simulink model "ofdm_txrx_supermimo"  (Simulink 7.1)
settings: Solver=VariableStepDiscrete  SolverMode=SingleTasking  StartTime=0.0  StopTime=simOnly_simLength  RelTol=1e-3
PostLoadFcn:
  | ofdm_rx_supermimo_init
InitFcn:
  | ofdm_rx_supermimo_init
StartFcn:
  | ofdm_rx_supermimo_init
values from neighbouring files (symbols this model uses):
  Action0_Config = 0* 31*2^18 + 0*2^24 + PHY_AUTORESPONSE_REQ_GOODHDR + PHY_AUTORESPONSE_REQ_GOODPKT + PHY_AUTORESPONSE_REQ_FLAGA    [ofdm_tx_supermimo_init.m]
  Action1_Config = 0* 62*2^18 + PHY_AUTORESPONSE_REQ_GOODHDR + PHY_AUTORESPONSE_REQ_GOODPKT    [ofdm_tx_supermimo_init.m]
  Action2_Config = 0    [ofdm_tx_supermimo_init.m]
  Action3_Config = 0    [ofdm_tx_supermimo_init.m]
  Action4_Config = 0    [ofdm_tx_supermimo_init.m]
  Action5_Config = 0    [ofdm_tx_supermimo_init.m]
  ActionID_AFTransmit = 31    [ofdm_tx_supermimo_init.m]
  ActionID_Disabled = 0    [ofdm_tx_supermimo_init.m]
  ActionID_SetFlagA = 62    [ofdm_tx_supermimo_init.m]
  ActionID_SetFlagB = 61    [ofdm_tx_supermimo_init.m]
  BER_RAM_init_size = 2048    [ofdm_tx_supermimo_init.m]
  CPLength = 16    [ofdm_tx_supermimo_init.m]
  longCorr_coef_i = [-4*real(LTS) 0]    [ofdm_rx_supermimo_init.m]
  longCorr_coef_q = [4*imag(LTS) 0]    [ofdm_rx_supermimo_init.m]
  Match0_Config = 0    [ofdm_tx_supermimo_init.m]
  Match1_Config = 0    [ofdm_tx_supermimo_init.m]
  Match2_Config = 0    [ofdm_tx_supermimo_init.m]
  Match3_Config = 0    [ofdm_tx_supermimo_init.m]
  Match4_Config = 0    [ofdm_tx_supermimo_init.m]
  Match5_Config = 0    [ofdm_tx_supermimo_init.m]
  Match6_Config = 0    [ofdm_tx_supermimo_init.m]
  Match7_Config = 0    [ofdm_tx_supermimo_init.m]
  max_CP_length = 16    [ofdm_tx_supermimo_init.m]
  max_num_baseRateSymbols = 31    [ofdm_tx_supermimo_init.m]
  max_num_subcarriers = 64    [ofdm_tx_supermimo_init.m]
  max_num_trainingSymbols = 15    [ofdm_tx_supermimo_init.m]
  max_numBytes = 16384    [ofdm_tx_supermimo_init.m]
  max_OFDM_symbols = 511    [ofdm_tx_supermimo_init.m]
  modConstellation_qam16 = .75*[1 3 -1 -3]./3    [ofdm_tx_supermimo_init.m]
  modConstellation_qam64 = 0.875*[3 1 5 7 -3 -1 -5 -7]./7    [ofdm_tx_supermimo_init.m]
  modConstellation_qpsk = [1 -1]./sqrt(2)    [ofdm_tx_supermimo_init.m]
  numBaseRateSymbols = ceil(numHeaderBytes / numBytes_BaseRateOFDMSymbol)    [ofdm_tx_supermimo_init.m]
  numSubcarriers = 64    [ofdm_tx_supermimo_init.m]
  numTrainingSymbols = 2    [ofdm_tx_supermimo_init.m]
  pilot_indicies = 7 + ( (64-21) * 2^8) + ( (64-7) * 2^16) + (21 * 2^24)    (= 356068103)    [ofdm_tx_supermimo_init.m]
  pilotValues = hex2dec('A57D') + (2^16 * hex2dec('5A82'))    [ofdm_tx_supermimo_init.m]
  pkt_crossCorr_thresh = 0.7    [ofdm_rx_supermimo_init.m]
  pkt_energy_thresh = 0    [ofdm_rx_supermimo_init.m]
  pktByteNums = numHeaderBytes +  				byteIndex_numPayloadBytes(1)*2^8 +  				byteIndex_numPayloadBytes(2)*2^16 +  				byteIndex_simpleDynModMasks * 2^24    [ofdm_rx_supermimo_init.m]
  PktDet_Delay = 58+32 +      Symbol_Timing_Offset*2^7 +      0*2^16 +      (117* 2^24)    (= 1962935642)    [ofdm_rx_supermimo_init.m]
  pktTiming_count_payloadStart = pktTiming_count_lsStart + pktTiming_ls_num*pktTiming_ls_length    [ofdm_rx_supermimo_init.m]
  preamble = preamble_scale*[shortsyms_10 longsyms_2]    [ofdm_tx_supermimo_init.m]
  preamble_ROM = preamble_I_ROM + preamble_Q_ROM*2^16    [ofdm_tx_supermimo_init.m]
  RAM_init_size = max_numBytes    (= 16384)    [ofdm_tx_supermimo_init.m]
  reg_AF_Tx_Blanking = (320+80) + ( (320+80+79)*2^16)    (= 31392144)    [ofdm_rx_supermimo_init.m]
  reg_AF_Tx_Scaling = 2944    [ofdm_rx_supermimo_init.m]
  reg_Interrupt_PktBuf_Control = reg_InterruptControl +  	1 * 2^16 +  	0 * 2^24    (= 65656)    [ofdm_rx_supermimo_init.m]
  reg_PilotCalcParams = (4 * 2^16) + (minPilotChanMag)    (= 262184.96)    [ofdm_rx_supermimo_init.m]
  regRx_chanEst_minMag = ((0.5)*2^15) + ((0.5 * 2^15)*2^16)    (= 1073758208)    [ofdm_rx_supermimo_init.m]
  regRx_coarseCalcCorrection = round((1.3e-3 - 1.2982e-3)*2^32)    [ofdm_rx_supermimo_init.m]
  regRx_pilotCalcCorrection = round(1.00115 * 2^31)    [ofdm_rx_supermimo_init.m]
  regRx_preCFOoptions = 0 * 2^0 +  	1 * 2^1 +  	0    (= 2)    [ofdm_rx_supermimo_init.m]
  rx_controlBits = 1 * 2  		 + 1 * 4  		 + 0 * 8  		 + 1 * 16 * rx_SISO_Mode  		 + 1 * 32  		 + 1 * 64  		 + 1 * 128  		 + 1 * 256  		 + 1 * 512  		 + 1 * 1024  		 + 1 * 4096  		 + 0 * 16384  		 + 0 * 32768  		 + 1 * 65536  		 + 0 * 131072  		 + 0 * 262144  		 + 1 * 524288  		 + 1 * 1048576 * tx_Alamouti_Mode  		 + 0 * 2097152           + 1 * 33554432  		 + 0 * 4194304  		 + 0 * 8388608  		 + 0 * 16777216  		 + 1 *  ...(+49 chars)    [ofdm_rx_supermimo_init.m]
  Rx_PktDet_LongCorr_Params = LongCorrCounterLoad + 0*2^8 + 0*2^16 + 255*2^24    (= 4278190325)    [ofdm_rx_supermimo_init.m]
  Rx_PktDet_LongCorrThresholds = 8000    [ofdm_rx_supermimo_init.m]
  rx_postEq_scaling = round(rxScaling*2^11) * (1 + 2^16)    [ofdm_rx_supermimo_init.m]
  RxDataScrambling_Seq = [40 198 78 63 82 173 102 245 48 111 172 115 147 230 216 93 72 65 62 2 205 242 122 90 128 83 105 97 73 10 5 252]    [ofdm_tx_supermimo_init.m]
  RxReg_FixedPktLen = 0    [ofdm_rx_supermimo_init.m]
  shortSymbol_time = shortSymbol_time(1:16).'    [ofdm_tx_supermimo_init.m]
  simOnly_numSamples = length(preamble)+( (numSubcarriers+CPLength)*(numTrainingSymbols + numBaseRateSymbols + numFullRateSymbols/2) )    [ofdm_tx_supermimo_init.m]
  train = [train train]    [ofdm_tx_supermimo_init.m]
  tx_controlBits = (antB_preambleShift * 2^4) + (2^1 * tx_Alamouti_Mode) +  	(2^0 * tx_SISO_Mode) + 4*0 + 8*1 + 0*256 + 512*0 + 1024*0 +  	0*hex2dec('1000') + 0*hex2dec('2000') + 0*hex2dec('4000') +  	1*hex2dec('8000') + 1*hex2dec('10000') + hex2dec('20000') +  	16*hex2dec('100000')    [ofdm_tx_supermimo_init.m]
  tx_delays = (50*2^0) + (0*2^8) + (30*2^12)    (= 122930)    [ofdm_tx_supermimo_init.m]
  tx_scaling = (3072) + (12288 * 2^16)    (= 805309440)    [ofdm_tx_supermimo_init.m]
  TxDataScrambling_Seq = [40 198 78 63 82 173 102 245 48 111 172 115 147 230 216 93 72 65 62 2 205 242 122 90 128 83 105 97 73 10 5 252]    [ofdm_tx_supermimo_init.m]
  txfilt_firpm = 0.95*txfilt_firpm./(max(txfilt_firpm))    [ofdm_tx_supermimo_init.m]
  txfilt_intfilt = [0 intfilt(4,4,.8)]    [ofdm_tx_supermimo_init.m]
  txReg_symbolCounts = (2^8 *numBaseRateSymbols ) + numTrainingSymbols    [ofdm_tx_supermimo_init.m]
  TxRx_FFTScaling = (3 * 2^0) +  	(2 * 2^2) +  	(1 * 2^4) +  	(1 * 2^6) +  	(1 * 2^8) +  	(0 * 2^10)    (= 347)    [ofdm_tx_supermimo_init.m]
undefined symbols (not defined in the file or in its folder): AntA, AntB, BaseRate, Down, gcbh, numBuffs, numBytes, Up, xlBlockLoadCallback, xlGetSimulinkPeriod, xlipgetpartsetting
libraries: xbsIndex_r4 x6035, simulink x4, dspsigops x4, commchan3 x2, dspsrcs4 x2, commrflib2 x2
(part 5 of 61)

== system ofdm_txrx_supermimo/Memory-mapped Registers/Static Parameters (might be registers someday) ==
  note: Replace with registers someday
      | to support dynamic transform and
      | cyclic prefix sizes
  Constant : xbsIndex_r4/Constant  arith_type=Unsigned  const=numSubcarriers  n_bits=ceil(log2(max_num_subcarriers)+1)  bin_pt=0  explicit_period=on  period=2  equ=P=C  opselect=C  inp2=PCIN>>17  opr=+  inp1=P  carry=CIN  dbl_ovrd=off  block_type=constant
      settings: block_version=VER_STRING_GOES_HERE
  Constant1 : xbsIndex_r4/Constant  arith_type=Unsigned  const=numSubcarriers+CPLength-1  n_bits=ceil(log2(max_num_subcarriers+CPLength)+1)  bin_pt=0  explicit_period=on  period=2  equ=P=C  opselect=C  inp2=PCIN>>17  opr=+  inp1=P  carry=CIN  dbl_ovrd=off  block_type=constant
      settings: block_version=VER_STRING_GOES_HERE
  Constant2 : xbsIndex_r4/Constant  arith_type=Unsigned  const=16  n_bits=5  bin_pt=0  explicit_period=on  period=1  equ=P=C  opselect=C  inp2=PCIN>>17  opr=+  inp1=P  carry=CIN  dbl_ovrd=off  block_type=constant
      settings: block_version=VER_STRING_GOES_HERE
  Down Sample8 : xbsIndex_r4/Down Sample  sample_ratio=2  sample_phase="Last Value of Frame  (most efficient)"  en=off  latency=1  dbl_ovrd=off  block_type=dsamp
      settings: block_version=VER_STRING_GOES_HERE
  tag scope: regRX_CP_Length=global, regRX_numSubcarriers=global, regRX_sampsPerSymMinusOne=global
  wires:
  Constant/1 -> [regRX_numSubcarriers]
  Constant1/1 -> [regRX_sampsPerSymMinusOne]
  Down Sample8/1 -> [regRX_CP_Length]
  Constant2/1 -> Down Sample8/1

== system ofdm_txrx_supermimo/Memory-mapped Registers/Tx Registers ==
  note: 0x2
  note: 0x1
  note: 0x0000_00FF - 8 bits
  note: 0x0000_0F00 - 4 bits
  note: 0x000F_F000 - 8 bits
  Control Bits Slices : Subsystem
  Down Sample1 : xbsIndex_r4/Down Sample  sample_ratio=2  sample_phase="Last Value of Frame  (most efficient)"  en=off  latency=1  dbl_ovrd=off  block_type=dsamp
      settings: block_version=VER_STRING_GOES_HERE
  Down Sample3 : xbsIndex_r4/Down Sample  sample_ratio=2  sample_phase="Last Value of Frame  (most efficient)"  en=off  latency=1  dbl_ovrd=off  block_type=dsamp
      settings: block_version=VER_STRING_GOES_HERE
  Down Sample4 : xbsIndex_r4/Down Sample  sample_ratio=4  sample_phase="Last Value of Frame  (most efficient)"  en=off  latency=1  dbl_ovrd=off  block_type=dsamp
      settings: block_version=VER_STRING_GOES_HERE
  Down Sample6 : xbsIndex_r4/Down Sample  sample_ratio=2  sample_phase="Last Value of Frame  (most efficient)"  en=off  latency=1  dbl_ovrd=off  block_type=dsamp
      settings: block_version=VER_STRING_GOES_HERE
  Read Only Register : Subsystem  AncestorBlock=|  mask{regName='Tx_PktRunning'}
      | WARP_Blockset/Register Wrappers
      | (Sysgen 10.1+)/Read Only Register
      mask prompts: regName "Register Name"
      mask help:
      | This block is a wrapper for System Generator's To Register shared memory block. This register is designed for use with WARP's sysgen2opb tool.
      |
      | Note: The Regisger Name paramter below must be unique for every instance of Read-Write and Read-Only registers in your system.
      mask init: same as ofdm_txrx_supermimo/Memory-mapped Registers/Rx Registers/Read Only Register
      settings: AttributesFormatString=RO Reg: %<regName>\n%<ReferenceBlock>
  Read-Write Register : Subsystem  AncestorBlock=|  mask{regName='Tx_Start_Reset_Control', out_dataType=Unsigned, out_numBits=32, out_binaryPt=0, initValue=0, sampPeriod=1}
      | WARP_Blockset/Register Wrappers
      | (Sysgen 10.1+)/Read-Write Register
      mask prompts: regName "Register Name"; out_dataType "Output Type"; out_numBits "Output Num Bits"; out_binaryPt "Output Binary Point"; initValue "Initial Value"; sampPeriod "Sample Period"
      mask help:
      | This block is a wrapper for two registers from the System Generator Shared Memory blockset:
      | a) A "From Register" block, which provides a write-only register for the host processor
      | b) A "To Register" block, which provides a read-only register for the host processor
      |
      | Note: The Regisger Name paramter below must be unique for every instance of shared-memory registers in your system. System Generator will throw an error if duplicate names are found.
      mask init: same as ofdm_txrx_supermimo/Memory-mapped Registers/AutoReply Registers/Read-Write Register1
      settings: AttributesFormatString=RW Reg: %<regName>
      mask StyleString: edit,popup(Unsigned|Signed  (2's comp)),edit,edit,edit,edit
  Read-Write Register1 : Subsystem  AncestorBlock=|  mask{regName='Tx_ControlBits', out_dataType=Unsigned, out_numBits=32, out_binaryPt=0, initValue=tx_controlBits, sampPeriod=1}
      | WARP_Blockset/Register Wrappers
      | (Sysgen 10.1+)/Read-Write Register
      mask prompts: regName "Register Name"; out_dataType "Output Type"; out_numBits "Output Num Bits"; out_binaryPt "Output Binary Point"; initValue "Initial Value"; sampPeriod "Sample Period"
      mask help:
      | This block is a wrapper for two registers from the System Generator Shared Memory blockset:
      | a) A "From Register" block, which provides a write-only register for the host processor
      | b) A "To Register" block, which provides a read-only register for the host processor
      |
      | Note: The Regisger Name paramter below must be unique for every instance of shared-memory registers in your system. System Generator will throw an error if duplicate names are found.
      mask init: same as ofdm_txrx_supermimo/Memory-mapped Registers/AutoReply Registers/Read-Write Register1
      settings: AttributesFormatString=RW Reg: %<regName>
      mask StyleString: edit,popup(Unsigned|Signed  (2's comp)),edit,edit,edit,edit
  Read-Write Register2 : Subsystem  AncestorBlock=|  mask{regName='Tx_Delays', out_dataType=Unsigned, out_numBits=32, out_binaryPt=0, initValue=tx_delays, sampPeriod=1}
      | WARP_Blockset/Register Wrappers
      | (Sysgen 10.1+)/Read-Write Register
      mask prompts: regName "Register Name"; out_dataType "Output Type"; out_numBits "Output Num Bits"; out_binaryPt "Output Binary Point"; initValue "Initial Value"; sampPeriod "Sample Period"
      mask help:
      | This block is a wrapper for two registers from the System Generator Shared Memory blockset:
      | a) A "From Register" block, which provides a write-only register for the host processor
      | b) A "To Register" block, which provides a read-only register for the host processor
      |
      | Note: The Regisger Name paramter below must be unique for every instance of shared-memory registers in your system. System Generator will throw an error if duplicate names are found.
      mask init: same as ofdm_txrx_supermimo/Memory-mapped Registers/AutoReply Registers/Read-Write Register1
      settings: AttributesFormatString=RW Reg: %<regName>
      mask StyleString: edit,popup(Unsigned|Signed  (2's comp)),edit,edit,edit,edit
  Reinterpret : xbsIndex_r4/Reinterpret  force_arith_type=on  arith_type=Unsigned  force_bin_pt=on  bin_pt=13  block_type=reinterpret
      settings: infoedit="Changes signal type without altering the binary representation.   You can changed the signal between signed and unsigned, and relocate the binary point.<P><P>Hardware notes: In hardware this block costs nothing.<P><P>Example:  Suppose the input is 6 bits wide, signed, with 2 fractional bits, and the output is forced to unsigned with 0 fractional bits.  Then an input of -2.0 (1110.00 in binary 2's complement) becomes an output of 56 (111000 in binary)."  block_version=10.1
  Reinterpret1 : xbsIndex_r4/Reinterpret  force_arith_type=on  arith_type=Unsigned  force_bin_pt=on  bin_pt=13  block_type=reinterpret
      settings: infoedit="Changes signal type without altering the binary representation.   You can changed the signal between signed and unsigned, and relocate the binary point.<P><P>Hardware notes: In hardware this block costs nothing.<P><P>Example:  Suppose the input is 6 bits wide, signed, with 2 fractional bits, and the output is forced to unsigned with 0 fractional bits.  Then an input of -2.0 (1110.00 in binary 2's complement) becomes an output of 56 (111000 in binary)."  block_version=10.1
  Slice1 : xbsIndex_r4/Slice  nbits=4  boolean_output=off  mode=Lower Bit Location + Width  bit1=0  base1=MSB of Input  bit0=8  base0=LSB of Input  dbl_ovrd=off  block_type=slice
      settings: infoedit="Extracts a given range of bits from each input sample and presents it at the output.  The output type is ordinarily unsigned with binary point at zero, but can be Boolean when the slice is one bit wide.<P><P>Hardware notes: In hardware this block costs nothing."  block_version=VER_STRING_GOES_HERE
  Slice10 : xbsIndex_r4/Slice  nbits=8  boolean_output=off  mode=Lower Bit Location + Width  bit1=0  base1=MSB of Input  bit0=0  base0=LSB of Input  dbl_ovrd=off  block_type=slice
      settings: infoedit="Extracts a given range of bits from each input sample and presents it at the output.  The output type is ordinarily unsigned with binary point at zero, but can be Boolean when the slice is one bit wide.<P><P>Hardware notes: In hardware this block costs nothing."  block_version=VER_STRING_GOES_HERE
  Slice14 : xbsIndex_r4/Slice  nbits=1  boolean_output=off  mode=Lower Bit Location + Width  bit1=0  base1=MSB of Input  bit0=0  base0=LSB of Input  dbl_ovrd=off  block_type=slice
      settings: infoedit="Extracts a given range of bits from each input sample and presents it at the output.  The output type is ordinarily unsigned with binary point at zero, but can be Boolean when the slice is one bit wide.<P><P>Hardware notes: In hardware this block costs nothing."  block_version=VER_STRING_GOES_HERE
  Slice15 : xbsIndex_r4/Slice  nbits=1  boolean_output=on  mode=Lower Bit Location + Width  bit1=0  base1=MSB of Input  bit0=1  base0=LSB of Input  dbl_ovrd=off  block_type=slice
      settings: infoedit="Extracts a given range of bits from each input sample and presents it at the output.  The output type is ordinarily unsigned with binary point at zero, but can be Boolean when the slice is one bit wide.<P><P>Hardware notes: In hardware this block costs nothing."  block_version=VER_STRING_GOES_HERE
  Slice2 : xbsIndex_r4/Slice  nbits=8  boolean_output=off  mode=Lower Bit Location + Width  bit1=0  base1=MSB of Input  bit0=12  base0=LSB of Input  dbl_ovrd=off  block_type=slice
      settings: infoedit="Extracts a given range of bits from each input sample and presents it at the output.  The output type is ordinarily unsigned with binary point at zero, but can be Boolean when the slice is one bit wide.<P><P>Hardware notes: In hardware this block costs nothing."  block_version=VER_STRING_GOES_HERE
  Slice7 : xbsIndex_r4/Slice  nbits=16  boolean_output=off  mode=Lower Bit Location + Width  bit1=0  base1=MSB of Input  bit0=0  base0=LSB of Input  dbl_ovrd=off  block_type=slice
      settings: infoedit="Extracts a given range of bits from each input sample and presents it at the output.  The output type is ordinarily unsigned with binary point at zero, but can be Boolean when the slice is one bit wide.<P><P>Hardware notes: In hardware this block costs nothing."  block_version=VER_STRING_GOES_HERE
  Slice8 : xbsIndex_r4/Slice  nbits=16  boolean_output=off  mode=Lower Bit Location + Width  bit1=0  base1=MSB of Input  bit0=16  base0=LSB of Input  dbl_ovrd=off  block_type=slice
      settings: infoedit="Extracts a given range of bits from each input sample and presents it at the output.  The output type is ordinarily unsigned with binary point at zero, but can be Boolean when the slice is one bit wide.<P><P>Hardware notes: In hardware this block costs nothing."  block_version=VER_STRING_GOES_HERE
  Write-Only Register1 : Subsystem  AncestorBlock=|  mask{regName='Tx_Scaling', out_dataType=Unsigned, out_numBits=32, out_binaryPt=0, initValue=tx_scaling, sampPeriod=1}
      | WARP_Blockset/Register Wrappers
      | (Sysgen 10.1+)/Write-Only Register
      mask prompts: regName "Register Name"; out_dataType "Output Type"; out_numBits "Output Num Bits"; out_binaryPt "Output Binary Point"; initValue "Initial Value"; sampPeriod "Sample Period"
      mask help:
      | This block is a wrapper for System Generator's "From Register" shared memory block.
      |
      | Note: The Regisger Name paramter below must be unique for every instance of shared-memory registers in your system. System Generator will throw an error if duplicate names are found.
      mask init: same as ofdm_txrx_supermimo/Memory-mapped Registers/Rx Registers/Write-Only Register
      settings: AttributesFormatString=RW Reg: %<regName>
      mask StyleString: edit,popup(Unsigned|Signed  (2's comp)),edit,edit,edit,edit
  tag scope: TxReg_ExtAutoTxEn_Delay=global, TxReg_PreambleScaling=global, TxReg_TxReset=global, TxReg_TxRunningOut_delay=global, TxReg_TxStart=global, TxReg_postIFFTScaling=global, regRx_AutoTx_ExtraDly=global
  wires:
  Read-Write Register1/1(D) -> Down Sample1/1
  Slice14/1 -> [TxReg_TxReset]
  Read-Write Register/1(D) -> Down Sample3/1
  Slice15/1 -> [TxReg_TxStart]
  Down Sample3/1 -> Slice14/1, Slice15/1
  [regTx_PktRunningEn] -> Read Only Register/2(En)
  Write-Only Register1/1(D) -> Slice8/1, Slice7/1
  Reinterpret/1 -> [TxReg_PreambleScaling]
  Slice7/1 -> Reinterpret/1
  Reinterpret1/1 -> [TxReg_postIFFTScaling]
  Slice8/1 -> Reinterpret1/1
  Down Sample1/1 -> Control Bits Slices/1(32b)
  [regTx_PktRunning] -> Read Only Register/1(D)
  Slice10/1 -> [TxReg_ExtAutoTxEn_Delay]
  Slice1/1 -> [regRx_AutoTx_ExtraDly]
  Slice2/1 -> [TxReg_TxRunningOut_delay]
  Read-Write Register2/1(D) -> Down Sample6/1, Down Sample4/1
  Down Sample6/1 -> Slice1/1, Slice10/1
  Down Sample4/1 -> Slice2/1

== system ofdm_txrx_supermimo/Memory-mapped Registers/TxRx Registers ==
  16LSB+8 : xbsIndex_r4/Slice  nbits=16  boolean_output=off  mode=Lower Bit Location + Width  bit1=0  base1=MSB of Input  bit0=8  base0=LSB of Input  dbl_ovrd=off  block_type=slice
      settings: infoedit="Extracts a given range of bits from each input sample and presents it at the output.  The output type is ordinarily unsigned with binary point at zero, but can be Boolean when the slice is one bit wide.<P><P>Hardware notes: In hardware this block costs nothing."  block_version=VER_STRING_GOES_HERE
  16LSB1 : xbsIndex_r4/Slice  nbits=ceil(log2(max_num_trainingSymbols))  boolean_output=off  mode=Lower Bit Location + Width  bit1=0  base1=MSB of Input  bit0=0  base0=LSB of Input  dbl_ovrd=off  block_type=slice
      settings: infoedit="Extracts a given range of bits from each input sample and presents it at the output.  The output type is ordinarily unsigned with binary point at zero, but can be Boolean when the slice is one bit wide.<P><P>Hardware notes: In hardware this block costs nothing."  block_version=VER_STRING_GOES_HERE
  16MSB1 : xbsIndex_r4/Slice  nbits=ceil(log2(max_num_baseRateSymbols))  boolean_output=off  mode=Lower Bit Location + Width  bit1=0  base1=MSB of Input  bit0=16  base0=LSB of Input  dbl_ovrd=off  block_type=slice
      settings: infoedit="Extracts a given range of bits from each input sample and presents it at the output.  The output type is ordinarily unsigned with binary point at zero, but can be Boolean when the slice is one bit wide.<P><P>Hardware notes: In hardware this block costs nothing."  block_version=VER_STRING_GOES_HERE
  6LSB+6 : xbsIndex_r4/Slice  nbits=6  boolean_output=off  mode=Lower Bit Location + Width  bit1=0  base1=MSB of Input  bit0=6  base0=LSB of Input  dbl_ovrd=off  block_type=slice
      settings: infoedit="Extracts a given range of bits from each input sample and presents it at the output.  The output type is ordinarily unsigned with binary point at zero, but can be Boolean when the slice is one bit wide.<P><P>Hardware notes: In hardware this block costs nothing."  block_version=VER_STRING_GOES_HERE
  6LSB1 : xbsIndex_r4/Slice  nbits=6  boolean_output=off  mode=Lower Bit Location + Width  bit1=0  base1=MSB of Input  bit0=0  base0=LSB of Input  dbl_ovrd=off  block_type=slice
      settings: infoedit="Extracts a given range of bits from each input sample and presents it at the output.  The output type is ordinarily unsigned with binary point at zero, but can be Boolean when the slice is one bit wide.<P><P>Hardware notes: In hardware this block costs nothing."  block_version=VER_STRING_GOES_HERE
  8LSB : xbsIndex_r4/Slice  nbits=8  boolean_output=off  mode=Lower Bit Location + Width  bit1=0  base1=MSB of Input  bit0=0  base0=LSB of Input  dbl_ovrd=off  block_type=slice
      settings: infoedit="Extracts a given range of bits from each input sample and presents it at the output.  The output type is ordinarily unsigned with binary point at zero, but can be Boolean when the slice is one bit wide.<P><P>Hardware notes: In hardware this block costs nothing."  block_version=VER_STRING_GOES_HERE
  AddSub1 : xbsIndex_r4/AddSub  mode=Addition  use_carryin=off  use_carryout=off  en=off  latency=0  precision=User Defined  arith_type=Unsigned  n_bits=1 + ceil(log2(max_num_trainingSymbols + max_num_baseRateSymbols))  bin_pt=0  quantization=Truncate  overflow=Wrap  dbl_ovrd=off  use_behavioral_HDL=on  pipelined=off  use_rpm=on  hw_selection=Fabric  block_type=addsub
      settings: xl_area=[0, 0, 0, 0, 0, 0, 0]  block_version=VER_STRING_GOES_HERE
  Down Sample{4,7,11,25} (4 alike) : xbsIndex_r4/Down Sample  sample_ratio=2  sample_phase="Last Value of Frame  (most efficient)"  en=off  latency=1  dbl_ovrd=off  block_type=dsamp
      settings: block_version=VER_STRING_GOES_HERE
  InterruptControl : Subsystem
  Read-Write Register : Subsystem  AncestorBlock=|  mask{regName='TxRx_Interrupt_PktBuf_Ctrl', out_dataType=Unsigned, out_numBits=32, out_binaryPt=0, initValue=reg_Interrupt_PktBuf_Control, sampPeriod=1}
      | WARP_Blockset/Register Wrappers
      | (Sysgen 10.1+)/Read-Write Register
      mask prompts: regName "Register Name"; out_dataType "Output Type"; out_numBits "Output Num Bits"; out_binaryPt "Output Binary Point"; initValue "Initial Value"; sampPeriod "Sample Period"
      mask help:
      | This block is a wrapper for two registers from the System Generator Shared Memory blockset:
      | a) A "From Register" block, which provides a write-only register for the host processor
      | b) A "To Register" block, which provides a read-only register for the host processor
      |
      | Note: The Regisger Name paramter below must be unique for every instance of shared-memory registers in your system. System Generator will throw an error if duplicate names are found.
      mask init: same as ofdm_txrx_supermimo/Memory-mapped Registers/AutoReply Registers/Read-Write Register1
      settings: AttributesFormatString=RW Reg: %<regName>
      mask StyleString: edit,popup(Unsigned|Signed  (2's comp)),edit,edit,edit,edit
  Read-Write Register2 : Subsystem  AncestorBlock=|  mask{regName='Tx_OFDM_SymCounts', out_dataType=Unsigned, out_numBits=32, out_binaryPt=0, initValue=txReg_symbolCounts, sampPeriod=1}
      | WARP_Blockset/Register Wrappers
      | (Sysgen 10.1+)/Read-Write Register
      mask prompts: regName "Register Name"; out_dataType "Output Type"; out_numBits "Output Num Bits"; out_binaryPt "Output Binary Point"; initValue "Initial Value"; sampPeriod "Sample Period"
      mask help:
      | This block is a wrapper for two registers from the System Generator Shared Memory blockset:
      | a) A "From Register" block, which provides a write-only register for the host processor
      | b) A "To Register" block, which provides a read-only register for the host processor
      |
      | Note: The Regisger Name paramter below must be unique for every instance of shared-memory registers in your system. System Generator will throw an error if duplicate names are found.
      mask init: same as ofdm_txrx_supermimo/Memory-mapped Registers/AutoReply Registers/Read-Write Register1
      settings: AttributesFormatString=RW Reg: %<regName>
      mask StyleString: edit,popup(Unsigned|Signed  (2's comp)),edit,edit,edit,edit
  Read-Write Register4 : Subsystem  AncestorBlock=|  mask{regName='Rx_OFDM_SymbolCounts', out_dataType=Unsigned, out_numBits=32, out_binaryPt=0, initValue=(2^16 * numBaseRateSymbols) + numTrainingSymbols, sampPeriod=1}
      | WARP_Blockset/Register Wrappers
      | (Sysgen 10.1+)/Read-Write Register
      mask prompts: regName "Register Name"; out_dataType "Output Type"; out_numBits "Output Num Bits"; out_binaryPt "Output Binary Point"; initValue "Initial Value"; sampPeriod "Sample Period"
      mask help:
      | This block is a wrapper for two registers from the System Generator Shared Memory blockset:
      | a) A "From Register" block, which provides a write-only register for the host processor
      | b) A "To Register" block, which provides a read-only register for the host processor
      |
      | Note: The Regisger Name paramter below must be unique for every instance of shared-memory registers in your system. System Generator will throw an error if duplicate names are found.
      mask init: same as ofdm_txrx_supermimo/Memory-mapped Registers/AutoReply Registers/Read-Write Register1
      settings: AttributesFormatString=RW Reg: %<regName>
      mask StyleString: edit,popup(Unsigned|Signed  (2's comp)),edit,edit,edit,edit
  Write-Only Register : Subsystem  AncestorBlock=|  mask{regName='TxRx_FFT_Scaling', out_dataType=Unsigned, out_numBits=12, out_binaryPt=0, initValue=TxRx_FFTScaling, sampPeriod=1}
      | WARP_Blockset/Register Wrappers
      | (Sysgen 10.1+)/Write-Only Register
      mask prompts: regName "Register Name"; out_dataType "Output Type"; out_numBits "Output Num Bits"; out_binaryPt "Output Binary Point"; initValue "Initial Value"; sampPeriod "Sample Period"
      mask help:
      | This block is a wrapper for System Generator's "From Register" shared memory block.
      |
      | Note: The Regisger Name paramter below must be unique for every instance of shared-memory registers in your system. System Generator will throw an error if duplicate names are found.
      mask init: same as ofdm_txrx_supermimo/Memory-mapped Registers/Rx Registers/Write-Only Register
      settings: AttributesFormatString=RW Reg: %<regName>
      mask StyleString: edit,popup(Unsigned|Signed  (2's comp)),edit,edit,edit,edit
  Write-Only Register2 : Subsystem  AncestorBlock=|  mask{regName='TxRx_Pilots_Index', out_dataType=Unsigned, out_numBits=32, out_binaryPt=0, initValue=pilot_indicies, sampPeriod=1}
      | WARP_Blockset/Register Wrappers
      | (Sysgen 10.1+)/Write-Only Register
      mask prompts: regName "Register Name"; out_dataType "Output Type"; out_numBits "Output Num Bits"; out_binaryPt "Output Binary Point"; initValue "Initial Value"; sampPeriod "Sample Period"
      mask help:
      | This block is a wrapper for System Generator's "From Register" shared memory block.
      |
      | Note: The Regisger Name paramter below must be unique for every instance of shared-memory registers in your system. System Generator will throw an error if duplicate names are found.
      mask init: same as ofdm_txrx_supermimo/Memory-mapped Registers/Rx Registers/Write-Only Register
      settings: AttributesFormatString=RW Reg: %<regName>
      mask StyleString: edit,popup(Unsigned|Signed  (2's comp)),edit,edit,edit,edit
  Write-Only Register4 : Subsystem  AncestorBlock=|  mask{regName='TxRx_Pilots_Values', out_dataType=Unsigned, out_numBits=32, out_binaryPt=0, initValue=pilotValues, sampPeriod=1}
      | WARP_Blockset/Register Wrappers
      | (Sysgen 10.1+)/Write-Only Register
      mask prompts: regName "Register Name"; out_dataType "Output Type"; out_numBits "Output Num Bits"; out_binaryPt "Output Binary Point"; initValue "Initial Value"; sampPeriod "Sample Period"
      mask help:
      | This block is a wrapper for System Generator's "From Register" shared memory block.
      |
      | Note: The Regisger Name paramter below must be unique for every instance of shared-memory registers in your system. System Generator will throw an error if duplicate names are found.
      mask init: same as ofdm_txrx_supermimo/Memory-mapped Registers/Rx Registers/Write-Only Register
      settings: AttributesFormatString=RW Reg: %<regName>
      mask StyleString: edit,popup(Unsigned|Signed  (2's comp)),edit,edit,edit,edit
  tag scope: Rx_FFTScaling=global, TxReg_OFDM_SymCounts=global, Tx_FFTScaling=global, regRx_numBaseRateSymbols=global, regRx_numTrainPlusBaseRateSyms=global, regRx_numTrainingSymbols=global, regTxRx_PilotToneIndex=global, regTxRx_PktBuffOffsets=global, regTx_PilotToneValues=global
  wires:
  Read-Write Register/1(D) -> Down Sample7/1, 16LSB+8/1
  Down Sample7/1 -> 8LSB/1
  Write-Only Register/1(D) -> Down Sample25/1
  Down Sample25/1 -> 6LSB1/1, 6LSB+6/1
  6LSB1/1 -> [Tx_FFTScaling]
  6LSB+6/1 -> [Rx_FFTScaling]
  16LSB+8/1 -> [regTxRx_PktBuffOffsets]
  8LSB/1 -> InterruptControl/1(Reg_8b)
  Write-Only Register2/1(D) -> Down Sample4/1
  Down Sample4/1 -> [regTxRx_PilotToneIndex]
  Write-Only Register4/1(D) -> [regTx_PilotToneValues]
  Read-Write Register2/1(D) -> [TxReg_OFDM_SymCounts]
  AddSub1/1 -> [regRx_numTrainPlusBaseRateSyms]
  16LSB1/1 -> [regRx_numTrainingSymbols], AddSub1/1
  16MSB1/1 -> [regRx_numBaseRateSymbols], AddSub1/2
  Read-Write Register4/1(D) -> Down Sample11/1
  Down Sample11/1 -> 16MSB1/1, 16LSB1/1

== system ofdm_txrx_supermimo/OFDM Rx MIMO/ADC Inputs & Antenna Selection ==
  note: Extra latency to cover delay through
      | MAX2829 amps -> ADC -> FPGA
  PktDetReset : Inport  OutDataType=sfix(16)  OutScaling=2^0
  "A I " : Inport  Port=2  OutDataType=sfix(16)  OutScaling=2^0
  "A Q " : Inport  Port=3  OutDataType=sfix(16)  OutScaling=2^0
  "B I " : Inport  Port=4  OutDataType=sfix(16)  OutScaling=2^0
  "B Q " : Inport  Port=5  OutDataType=sfix(16)  OutScaling=2^0
  Antenna Selection : Subsystem
  Constant4 : Constant  OutDataType=sfix(16)  OutScaling=2^0
  Constant5 : Constant  OutDataType=sfix(16)  OutScaling=2^0
  Convert : xbsIndex_r4/Convert  arith_type=Boolean  n_bits=16  bin_pt=14  quantization=Truncate  overflow=Wrap  latency=0  dbl_ovrd=off  pipeline=off  block_type=convert
      settings: block_version=10.1.3
  Convert1 : xbsIndex_r4/Convert  arith_type=Boolean  n_bits=16  bin_pt=14  quantization=Truncate  overflow=Wrap  latency=0  dbl_ovrd=off  pipeline=off  block_type=convert
      settings: block_version=10.1.3
  Down Sample{,1..3} (4 alike) : xbsIndex_r4/Down Sample  sample_ratio=4  sample_phase="Last Value of Frame  (most efficient)"  en=off  latency=1  dbl_ovrd=off  block_type=dsamp
      settings: block_version=8.2.02
  Down Sample6 : xbsIndex_r4/Down Sample  sample_ratio=4  sample_phase="Last Value of Frame  (most efficient)"  en=off  latency=4  dbl_ovrd=off  block_type=dsamp
      settings: block_version=8.2.02
  Down Sample9 : xbsIndex_r4/Down Sample  sample_ratio=4  sample_phase="Last Value of Frame  (most efficient)"  en=off  latency=4  dbl_ovrd=off  block_type=dsamp
      settings: block_version=8.2.02
  Inverter : xbsIndex_r4/Inverter  en=off  latency=0  dbl_ovrd=off  block_type=inv
  Inverter1 : xbsIndex_r4/Inverter  en=off  latency=0  dbl_ovrd=off  block_type=inv
  Inverter2 : xbsIndex_r4/Inverter  en=off  latency=0  dbl_ovrd=off  block_type=inv
  Logical1 : xbsIndex_r4/Logical  logical_function=AND  inputs=2  en=off  latency=0  precision=Full  arith_type=Unsigned  n_bits=8  bin_pt=2  align_bp=on  dbl_ovrd=off  block_type=logical
      settings: xl_area=[1 0 0 1 0 0 0]  block_version=VER_STRING_GOES_HERE
  Logical2 : xbsIndex_r4/Logical  logical_function=OR  inputs=2  en=off  latency=0  precision=Full  arith_type=Unsigned  n_bits=8  bin_pt=2  align_bp=on  dbl_ovrd=off  block_type=logical
      settings: xl_area=[1 0 0 1 0 0 0]  block_version=VER_STRING_GOES_HERE
  Logical3 : xbsIndex_r4/Logical  logical_function=AND  inputs=2  en=off  latency=0  precision=Full  arith_type=Unsigned  n_bits=8  bin_pt=2  align_bp=on  dbl_ovrd=off  block_type=logical
      settings: xl_area=[1 0 0 1 0 0 0]  block_version=VER_STRING_GOES_HERE
  Register{,1..3} (4 alike) : xbsIndex_r4/Register  init=0  rst=on  en=off  dbl_ovrd=off  block_type=register
  Rx_AntA_ADCI : xbsIndex_r4/Gateway In  arith_type="Signed  (2's comp)"  n_bits=14  bin_pt=13  quantization="Round  (unbiased: +/- Inf)"  overflow=Saturate  period=1  dbl_ovrd=off  timing_constraint=None  locs_specified=off  LOCs={}  block_type=gatewayin
      settings: infoedit="Gateway in block.  Converts inputs of type Simulink integer, double and fixed point to  Xilinx fixed point type.<P><P>Hardware notes:  In hardware these blocks become top level input ports."  block_version=VER_STRING_GOES_HERE
  Rx_AntA_ADCQ : xbsIndex_r4/Gateway In  arith_type="Signed  (2's comp)"  n_bits=14  bin_pt=13  quantization="Round  (unbiased: +/- Inf)"  overflow=Saturate  period=1  dbl_ovrd=off  timing_constraint=None  locs_specified=off  LOCs={}  block_type=gatewayin
      settings: infoedit="Gateway in block.  Converts inputs of type Simulink integer, double and fixed point to  Xilinx fixed point type.<P><P>Hardware notes:  In hardware these blocks become top level input ports."  block_version=VER_STRING_GOES_HERE
  Rx_AntA_AGC_Done : xbsIndex_r4/Gateway In  arith_type=Unsigned  n_bits=1  bin_pt=0  quantization="Round  (unbiased: +/- Inf)"  overflow=Saturate  period=1  dbl_ovrd=off  timing_constraint=None  locs_specified=off  LOCs={}  block_type=gatewayin
      settings: infoedit="Gateway in block.  Converts inputs of type Simulink integer, double and fixed point to  Xilinx fixed point type.<P><P>Hardware notes:  In hardware these blocks become top level input ports."  block_version=VER_STRING_GOES_HERE
  Rx_AntB_ADCI : xbsIndex_r4/Gateway In  arith_type="Signed  (2's comp)"  n_bits=14  bin_pt=13  quantization="Round  (unbiased: +/- Inf)"  overflow=Saturate  period=1  dbl_ovrd=off  timing_constraint=None  locs_specified=off  LOCs={}  block_type=gatewayin
      settings: infoedit="Gateway in block.  Converts inputs of type Simulink integer, double and fixed point to  Xilinx fixed point type.<P><P>Hardware notes:  In hardware these blocks become top level input ports."  block_version=VER_STRING_GOES_HERE
  Rx_AntB_ADCQ : xbsIndex_r4/Gateway In  arith_type="Signed  (2's comp)"  n_bits=14  bin_pt=13  quantization="Round  (unbiased: +/- Inf)"  overflow=Saturate  period=1  dbl_ovrd=off  timing_constraint=None  locs_specified=off  LOCs={}  block_type=gatewayin
      settings: infoedit="Gateway in block.  Converts inputs of type Simulink integer, double and fixed point to  Xilinx fixed point type.<P><P>Hardware notes:  In hardware these blocks become top level input ports."  block_version=VER_STRING_GOES_HERE
  Rx_AntB_AGC_Done : xbsIndex_r4/Gateway In  arith_type=Unsigned  n_bits=1  bin_pt=0  quantization="Round  (unbiased: +/- Inf)"  overflow=Saturate  period=1  dbl_ovrd=off  timing_constraint=None  locs_specified=off  LOCs={}  block_type=gatewayin
      settings: infoedit="Gateway in block.  Converts inputs of type Simulink integer, double and fixed point to  Xilinx fixed point type.<P><P>Hardware notes:  In hardware these blocks become top level input ports."  block_version=VER_STRING_GOES_HERE
  A I : Outport  OutDataType=sfix(16)  OutScaling=2^0
  A Q : Outport  Port=2  OutDataType=sfix(16)  OutScaling=2^0
  B I : Outport  Port=3  OutDataType=sfix(16)  OutScaling=2^0
  B Q : Outport  Port=4  OutDataType=sfix(16)  OutScaling=2^0
  tag scope: AntA_ADC_I_Filt=global, AntA_ADC_Q_Filt=global, AntA_AGC_Done=global, AntB_AGC_Done=global
  wires:
  "B Q "/1 -> Rx_AntB_ADCQ/1
  "B I "/1 -> Rx_AntB_ADCI/1
  "A Q "/1 -> Rx_AntA_ADCQ/1
  "A I "/1 -> Rx_AntA_ADCI/1
  Down Sample/1 -> Antenna Selection/1(AntA_I)
  Down Sample1/1 -> Antenna Selection/2(AntA_Q)
  Down Sample2/1 -> Antenna Selection/3(AntB_I)
  Down Sample3/1 -> Antenna Selection/4(AntB_Q)
  Antenna Selection/1(A I) -> [AntA_ADC_I_Filt], Register/1
  Antenna Selection/2(A Q) -> [AntA_ADC_Q_Filt], Register1/1
  PktDetReset/1 -> Antenna Selection/5(PktDetReset)
  Constant5/1 -> Rx_AntB_AGC_Done/1
  Rx_AntB_AGC_Done/1 -> Down Sample9/1
  Down Sample9/1 -> [AntB_AGC_Done], Convert1/1
  Constant4/1 -> Rx_AntA_AGC_Done/1
  Rx_AntA_AGC_Done/1 -> Down Sample6/1
  Down Sample6/1 -> [AntA_AGC_Done], Convert/1
  Rx_AntA_ADCI/1 -> Down Sample/1
  Rx_AntA_ADCQ/1 -> Down Sample1/1
  Rx_AntB_ADCI/1 -> Down Sample2/1
  Rx_AntB_ADCQ/1 -> Down Sample3/1
  Register/1 -> A I/1
  Register1/1 -> A Q/1
  Register2/1 -> B I/1
  Register3/1 -> B Q/1
  Antenna Selection/4(B Q) -> Register3/1
  Antenna Selection/3(B I) -> Register2/1
  Inverter1/1 -> Register2/2, Register3/2, Logical3/2
  Convert/1 -> Inverter/1
  Convert1/1 -> Inverter1/1
  Inverter/1 -> Logical1/2
  Inverter2/1 -> Logical1/1
  Antenna Selection/5(A_swapped) -> Inverter2/1, Logical3/1
  Logical1/1 -> Logical2/1
  Logical3/1 -> Logical2/2
  Logical2/1 -> Register1/2, Register/2

== system ofdm_txrx_supermimo/OFDM Rx MIMO/AF Buffers ==
  PktDone : Inport  OutDataType=sfix(16)  OutScaling=2^0
  Payload : Inport  Port=2  OutDataType=sfix(16)  OutScaling=2^0
  AntA_I : Inport  Port=3  OutDataType=sfix(16)  OutScaling=2^0
  AntA_Q : Inport  Port=4  OutDataType=sfix(16)  OutScaling=2^0
  AF Buffers : Subsystem
  tag scope: AF_OutputSamples_I=global, AF_OutputSamples_Q=global, AF_PreambleIndex=global, AF_TxDone=global
  wires:
  AntA_I/1 -> AF Buffers/1(AntA_I)
  AntA_Q/1 -> AF Buffers/2(AntA_Q)
  Payload/1 -> AF Buffers/3(Payload)
  PktDone/1 -> AF Buffers/4(PktDone)
  AF Buffers/1(I) -> [AF_OutputSamples_I]
  AF Buffers/2(Q) -> [AF_OutputSamples_Q]
  AF Buffers/3(AF Pream Index) -> [AF_PreambleIndex]
  AF Buffers/4(AFTx Done) -> [AF_TxDone]

== system ofdm_txrx_supermimo/OFDM Rx MIMO/ChipScope ==
  ChipScope1 : xbsIndex_r4/ChipScope  num_trig_ports=5  current_port=4  show_trig_port=4  match_units=1  match_type=Basic with edges  data_is_trigger=off  num_data_ports=18  data_depth=4096  SRL16s=on  match_type_t0=2  match_type_t1=2  match_type_t2=2  match_type_t3=2  match_type_t4=2  match_type_t5=1  match_type_t6=1  match_type_t7=1  match_type_t8=1  match_type_t9=1  match_type_t10=1  match_type_t11=1  match_type_t12=1  match_type_t13=1  match_type_t14=1  match_type_t15=1  match_units_t0=1  match_units_t1=1  match_units_t2=1  match_units_t3=1  match_units_t4=1  match_units_t5=1  match_units_t6=1  match_units_t7=1  match_units_t8=1  match_units_t9=1  match_units_t10=1  match_units_t11=1  match_units_t12=1  match_units_t13=1  match_units_t14=1  match_units_t15=1  dbl_ovrd=0  block_type=chipscope
      settings: infoedit="Enables run-time debugging and verification of signals by inserting ChipScope Pro ICON and ILA cores.<br><br>  Restrictions: <br>Only one ChipScope core can be instantiated in a System Generator design.This block cannot be used at the same time as JTAG hardware co-simulation.A design or subsystem containing a ChipScope block must have at least one output port."  block_version=VER_STRING_GOES_HERE
  Constant1 : Constant  Value=0  OutDataType=sfix(16)  OutScaling=2^0
  Convert1 : xbsIndex_r4/Convert  arith_type="Signed  (2's comp)"  n_bits=14  bin_pt=12  quantization=Truncate  overflow=Saturate  latency=0  dbl_ovrd=off  pipeline=off  block_type=convert
      settings: xl_area=[0 0 0 0 0 0 0]  block_version=VER_STRING_GOES_HERE
  Convert2 : xbsIndex_r4/Convert  arith_type=Unsigned  n_bits=14  bin_pt=0  quantization=Truncate  overflow=Wrap  latency=0  dbl_ovrd=off  pipeline=off  block_type=convert
      settings: xl_area=[0 0 0 0 0 0 0]  block_version=VER_STRING_GOES_HERE
  Counter1 : xbsIndex_r4/Counter  cnt_type=Free Running  cnt_to=Inf  operation=Up  start_count=0  cnt_by_val=1  arith_type=Unsigned  n_bits=2  bin_pt=0  load_pin=off  rst=off  en=off  explicit_period=on  period=1  dbl_ovrd=off  use_behavioral_HDL=off  use_rpm=on  implementation=Fabric  block_type=counter
      settings: xl_area=[5 10 0 10 0 0 0]  block_version=VER_STRING_GOES_HERE
  Slice2 : xbsIndex_r4/Slice  nbits=1  boolean_output=off  mode=Upper Bit Location + Width  bit1=0  base1=MSB of Input  bit0=0  base0=LSB of Input  dbl_ovrd=off  block_type=slice
      settings: infoedit="Extracts a given range of bits from each input sample and presents it at the output.  The output type is ordinarily unsigned with binary point at zero, but can be Boolean when the slice is one bit wide.<P><P>Hardware notes: In hardware this block costs nothing."  block_version=VER_STRING_GOES_HERE
  Up Sample{1..3,12,14,17..18} (7 alike) : xbsIndex_r4/Up Sample  sample_ratio=2  copy_samples=on  dbl_ovrd=off  block_type=usamp
      settings: infoedit="Up samples input data.  Inserted values  can be zeros or copies of the most recent input sample.<P><P>Hardware notes: No hardware is needed if inserted values are copies of the input sample; otherwise, a mux and single bit flip-flop are used."  block_version=VER_STRING_GOES_HERE
  Up Sample{4..7,11,15..16,21} (8 alike) : xbsIndex_r4/Up Sample  sample_ratio=4  copy_samples=on  dbl_ovrd=off  block_type=usamp
      settings: infoedit="Up samples input data.  Inserted values  can be zeros or copies of the most recent input sample.<P><P>Hardware notes: No hardware is needed if inserted values are copies of the input sample; otherwise, a mux and single bit flip-flop are used."  block_version=VER_STRING_GOES_HERE
  debug_chipscopeTrig1 : xbsIndex_r4/Gateway In  arith_type=Boolean  n_bits=14  bin_pt=13  quantization="Round  (unbiased: +/- Inf)"  overflow=Saturate  period=1  dbl_ovrd=off  timing_constraint=None  locs_specified=off  LOCs={}  block_type=gatewayin
      settings: infoedit="Gateway in block.  Converts inputs of type Simulink integer, double and fixed point to  Xilinx fixed point type.<P><P>Hardware notes:  In hardware these blocks become top level input ports."  block_version=VER_STRING_GOES_HERE
  wires:
  [Payload] -> Up Sample11/1
  [AntA_ADC_I_Filt] -> Up Sample21/1
  [EQ_I] -> Up Sample17/1
  [EQ_Q] -> Up Sample18/1
  [Long_Corr] -> Convert2/1
  Constant1/1 -> debug_chipscopeTrig1/1
  Up Sample11/1 -> ChipScope1/1, ChipScope1/6  "Payload"
  Up Sample16/1 -> ChipScope1/13  "LongCorr"
  Up Sample17/1 -> ChipScope1/11  "EQ_I"
  Up Sample18/1 -> ChipScope1/12  "EQ_Q"
  Up Sample21/1 -> ChipScope1/9  "AntA_ADC_I"
  debug_chipscopeTrig1/1 -> ChipScope1/2  "ExtTrig"
  [intOut_GoodPkt] -> Up Sample12/1
  [intOut_BadPkt] -> Up Sample14/1
  Up Sample12/1 -> ChipScope1/3, ChipScope1/7  "Good Pkt Int"
  Up Sample14/1 -> ChipScope1/4, ChipScope1/8  "Bad Pkt Int"
  [AntA_ADC_Q_Filt] -> Up Sample15/1
  Up Sample15/1 -> ChipScope1/10  "AntA_ADC_Q"
  Counter1/1 -> Slice2/1
  Slice2/1 -> ChipScope1/5  "SampClk"
  [Chipscope_ChanEst] -> ChipScope1/15  "ChanEst"
  [PilotPhaseErr] -> ChipScope1/16  "PilotPhaseErr"
  Convert2/1 -> Up Sample16/1
  [intOut_GoodHeader] -> Up Sample2/1
  [intOut_BadHeader] -> Up Sample3/1
  [DebugOut_EVM] -> Convert1/1
  Up Sample1/1 -> ChipScope1/14  "EVM"
  Up Sample2/1 -> ChipScope1/17  "Good Header Int"
  Up Sample3/1 -> ChipScope1/18  "Bad Header Int"
  Up Sample4/1 -> ChipScope1/19  "PktDet"
  [pktDet] -> Up Sample4/1
  [AntA_AGC_Done] -> Up Sample5/1
  [AntA_GainBB] -> Up Sample6/1
  [AntA_GainRF] -> Up Sample7/1
  Up Sample5/1 -> ChipScope1/20  "AntA_AGC_Done"
  Up Sample6/1 -> ChipScope1/21  "AntA_GainBB"
  Up Sample7/1 -> ChipScope1/22  "AntA_GainRF"
  [FFT_Overflow] -> ChipScope1/23  "FFT_Overflow"
  Convert1/1 -> Up Sample1/1

== system ofdm_txrx_supermimo/OFDM Rx MIMO/Coarse Freq Correction ==
  Reset : Inport  OutDataType=sfix(16)  OutScaling=2^0
  PktDet : Inport  Port=2  OutDataType=sfix(16)  OutScaling=2^0
  AntA I : Inport  Port=3  OutDataType=sfix(16)  OutScaling=2^0
  AntA Q : Inport  Port=4  OutDataType=sfix(16)  OutScaling=2^0
  AntB I : Inport  Port=5  OutDataType=sfix(16)  OutScaling=2^0
  AntB Q : Inport  Port=6  OutDataType=sfix(16)  OutScaling=2^0
  A*B : Subsystem
  A*B1 : Subsystem
  Bus Creator1 : BusCreator  Inputs=2
  CFO : Scope  NumInputPorts=5
      settings: TimeRange=14608  YMin=-0.25~-0.25~-1~-1~0  YMax=0.2~0.25~1~1~1  SaveName=ScopeData26
  Coarse CFO Calc : Subsystem
  Complex to Magnitude-Angle : ComplexToMagnitudeAngle
  Complex to Real-Imag : ComplexToRealImag
  Convert{,1..3} (4 alike) : xbsIndex_r4/Convert  arith_type="Signed  (2's comp)"  n_bits=16  bin_pt=15  quantization=Truncate  overflow=Flag as error  latency=0  dbl_ovrd=off  pipeline=off  block_type=convert
      settings: xl_area=[0 0 0 0 0 0 0]  block_version=VER_STRING_GOES_HERE
  DDS : Subsystem
  Gateway Out{,1..3} (4 alike) : xbsIndex_r4/Gateway Out  hdl_port=off  timing_constraint=None  locs_specified=off  LOCs={}  block_type=gatewayout
      settings: infoedit="Gateway out block.  Converts Xilinx fixed point inputs into ouputs of type Simulink integer, double, or fixed point.<P><P>Hardware notes:  In hardware these blocks become top level output ports or are discarded, depending on how they are configured."  xl_area=[0 0 0 0 0 0 0]  block_version=VER_STRING_GOES_HERE
  Mux2 : Mux  Inputs=2
  PreSpin CFO Sel : Subsystem
  Product : Product  OutDataType=sfix(16)  OutScaling=2^0  RndMeth=Zero
  Real-Imag to Complex : RealImagToComplex
  "AntA I " : Outport  OutDataType=sfix(16)  OutScaling=2^0
  "AntA Q " : Outport  Port=2  OutDataType=sfix(16)  OutScaling=2^0
  "AntB I " : Outport  Port=3  OutDataType=sfix(16)  OutScaling=2^0
  "AntB Q " : Outport  Port=4  OutDataType=sfix(16)  OutScaling=2^0
  tag scope: CFO_PhaseInc=global
  wires:
  A*B/1(Re) -> Convert/1
  A*B/2(Im1) -> Convert1/1
  A*B1/1(Re) -> Convert2/1
  A*B1/2(Im1) -> Convert3/1
  DDS/1(Q) -> Gateway Out/1, A*B1/2(Im[B]), A*B/3(Im[B])
  DDS/2(I) -> Gateway Out1/1, A*B1/1(Re[B]), A*B/4(Re[B])
  Gateway Out1/1 -> Mux2/1, Real-Imag to Complex/1
  Gateway Out/1 -> Mux2/2, Real-Imag to Complex/2
  Mux2/1 -> CFO/3
  Bus Creator1/1 -> CFO/4
  [freqOffset] -> Complex to Real-Imag/1, Product/2
  Complex to Real-Imag/1 -> Bus Creator1/1
  Complex to Real-Imag/2 -> Bus Creator1/2
  Gateway Out2/1 -> CFO/2
  Gateway Out3/1 -> CFO/1
  AntB I/1 -> A*B1/3(Re[A])
  AntB Q/1 -> A*B1/4(Im[A])
  AntA I/1 -> A*B/1(Re[A]), Coarse CFO Calc/1(I In)
  AntA Q/1 -> A*B/2(Im[A]), Coarse CFO Calc/2(Q In)
  Convert/1 -> "AntA I "/1
  Convert1/1 -> "AntA Q "/1
  Real-Imag to Complex/1 -> Product/1
  Product/1 -> Complex to Magnitude-Angle/1
  Complex to Magnitude-Angle/2 -> CFO/5
  Convert2/1 -> "AntB I "/1
  Convert3/1 -> "AntB Q "/1
  Reset/1 -> Coarse CFO Calc/4(Reset), DDS/3(Rst), PreSpin CFO Sel/1(Rst)
  PktDet/1 -> Gateway Out2/1, Coarse CFO Calc/3(RxPkt Active)
  Coarse CFO Calc/1(Phase Inc) -> Gateway Out3/1, [CFO_PhaseInc], DDS/2(Rx_CoarseEst)
  PreSpin CFO Sel/1(Tx_PhaseInc) -> DDS/1(Tx_PhaseInc)
